simulink model "large_model"  (Simulink 8.0)
settings: Solver=ode45  StartTime=0.0  StopTime=10.0  RelTol=1e-3
(part 17 of 21)

== system large_model/RDC4002_1/RDC Hardware ==
  In : Inport
  PWR_In : Inport  Port=2
  Out : Outport
  wires:
  In/1 -> Out/1

== system large_model/RDC4002_2/RDC Hardware ==
  In : Inport
  PWR_In : Inport  Port=2
  Out : Outport
  wires:
  In/1 -> Out/1

== system large_model/RDC4002_3/RDC Hardware ==
  In : Inport
  PWR_In : Inport  Port=2
  Out : Outport
  wires:
  In/1 -> Out/1

== system large_model/RDC4002_4/RDC Hardware ==
  In : Inport
  PWR_In : Inport  Port=2
  Out : Outport
  wires:
  In/1 -> Out/1

== system large_model/GPM_IO_1/IO_Card/IO Card Hardware ==
  In : Inport
  PWR : Inport  Port=2
  Out : Outport
  wires:
  In/1 -> Out/1

== system large_model/GPM_IO_1/Integrated_GPM0/End_System ==
  From_GPM : Inport
  From_Switch_ChA : Inport  Port=2
  From_Switch_ChB : Inport  Port=3
  PWR : Inport  Port=4
  Bus Creator : BusCreator  Inputs=2
  ES Hardware : Subsystem
  To_GPM : Outport
  To_Switch_ChA : Outport  Port=2
  To_Switch_ChB : Outport  Port=3
  wires:
  From_GPM/1 -> ES Hardware/1(In1)
  Bus Creator/1 -> ES Hardware/2(In2)
  PWR/1 -> ES Hardware/3(PWR)
  ES Hardware/2(Out2) -> To_GPM/1
  ES Hardware/1(Out1) -> To_Switch_ChB/1, To_Switch_ChA/1
  From_Switch_ChB/1 -> Bus Creator/2  "Switch_ChB"
  From_Switch_ChA/1 -> Bus Creator/1  "Switch_ChA"

== system large_model/GPM_IO_1/Integrated_GPM0/GPM ==
  note: Este componente foi colocado devido a uma limitação do EFTG. Na versão 1.2.1,
      | não é permitido fazer uma conexão direta entre a entrada e a saída.
  From_IO : Inport
  PWR_In : Inport  Port=2
  GPM Hardware : Subsystem
  PWR : Subsystem
  To_ES : Outport
  PWR_Out : Outport  Port=2
  wires:
  PWR_In/1 -> GPM Hardware/2(PWR_In), PWR/1(In)
  GPM Hardware/1(Out) -> To_ES/1
  PWR/1(Out) -> PWR_Out/1
  From_IO/1 -> GPM Hardware/1(In)

== system large_model/GPM_IO_2/IO_Card/IO Card Hardware ==
  In : Inport
  PWR : Inport  Port=2
  Out : Outport
  wires:
  In/1 -> Out/1

== system large_model/GPM_IO_2/Integrated_GPM0/End_System ==
  From_GPM : Inport
  From_Switch_ChA : Inport  Port=2
  From_Switch_ChB : Inport  Port=3
  PWR : Inport  Port=4
  Bus Creator : BusCreator  Inputs=2
  ES Hardware : Subsystem
  To_GPM : Outport
  To_Switch_ChA : Outport  Port=2
  To_Switch_ChB : Outport  Port=3
  wires:
  From_GPM/1 -> ES Hardware/1(In1)
  Bus Creator/1 -> ES Hardware/2(In2)
  PWR/1 -> ES Hardware/3(PWR)
  ES Hardware/2(Out2) -> To_GPM/1
  ES Hardware/1(Out1) -> To_Switch_ChB/1, To_Switch_ChA/1
  From_Switch_ChB/1 -> Bus Creator/2  "Switch_ChB"
  From_Switch_ChA/1 -> Bus Creator/1  "Switch_ChA"

== system large_model/GPM_IO_2/Integrated_GPM0/GPM ==
  note: Este componente foi colocado devido a uma limitação do EFTG. Na versão 1.2.1,
      | não é permitido fazer uma conexão direta entre a entrada e a saída.
  From_IO : Inport
  PWR_In : Inport  Port=2
  GPM Hardware : Subsystem
  PWR : Subsystem
  To_ES : Outport
  PWR_Out : Outport  Port=2
  wires:
  PWR_In/1 -> GPM Hardware/2(PWR_In), PWR/1(In)
  GPM Hardware/1(Out) -> To_ES/1
  PWR/1(Out) -> PWR_Out/1
  From_IO/1 -> GPM Hardware/1(In)

== system large_model/GPM_IO_3/IO_Card/IO Card Hardware ==
  In : Inport
  PWR : Inport  Port=2
  Out : Outport
  wires:
  In/1 -> Out/1

== system large_model/GPM_IO_3/Integrated_GPM0/End_System ==
  From_GPM : Inport
  From_Switch_ChA : Inport  Port=2
  From_Switch_ChB : Inport  Port=3
  PWR : Inport  Port=4
  Bus Creator : BusCreator  Inputs=2
  ES Hardware : Subsystem
  To_GPM : Outport
  To_Switch_ChA : Outport  Port=2
  To_Switch_ChB : Outport  Port=3
  wires:
  From_GPM/1 -> ES Hardware/1(In1)
  Bus Creator/1 -> ES Hardware/2(In2)
  PWR/1 -> ES Hardware/3(PWR)
  ES Hardware/2(Out2) -> To_GPM/1
  ES Hardware/1(Out1) -> To_Switch_ChB/1, To_Switch_ChA/1
  From_Switch_ChB/1 -> Bus Creator/2  "Switch_ChB"
  From_Switch_ChA/1 -> Bus Creator/1  "Switch_ChA"

== system large_model/GPM_IO_3/Integrated_GPM0/GPM ==
  note: Este componente foi colocado devido a uma limitação do EFTG. Na versão 1.2.1,
      | não é permitido fazer uma conexão direta entre a entrada e a saída.
  From_IO : Inport
  PWR_In : Inport  Port=2
  GPM Hardware : Subsystem
  PWR : Subsystem
  To_ES : Outport
  PWR_Out : Outport  Port=2
  wires:
  PWR_In/1 -> GPM Hardware/2(PWR_In), PWR/1(In)
  GPM Hardware/1(Out) -> To_ES/1
  PWR/1(Out) -> PWR_Out/1
  From_IO/1 -> GPM Hardware/1(In)

== system large_model/GPM_IO_4/IO_Card/IO Card Hardware ==
  In : Inport
  PWR : Inport  Port=2
  Out : Outport
  wires:
  In/1 -> Out/1

== system large_model/GPM_IO_4/Integrated_GPM0/End_System ==
  From_GPM : Inport
  From_Switch_ChA : Inport  Port=2
  From_Switch_ChB : Inport  Port=3
  PWR : Inport  Port=4
  Bus Creator : BusCreator  Inputs=2
  ES Hardware : Subsystem
  To_GPM : Outport
  To_Switch_ChA : Outport  Port=2
  To_Switch_ChB : Outport  Port=3
  wires:
  From_GPM/1 -> ES Hardware/1(In1)
  Bus Creator/1 -> ES Hardware/2(In2)
  PWR/1 -> ES Hardware/3(PWR)
  ES Hardware/2(Out2) -> To_GPM/1
  ES Hardware/1(Out1) -> To_Switch_ChB/1, To_Switch_ChA/1
  From_Switch_ChB/1 -> Bus Creator/2  "Switch_ChB"
  From_Switch_ChA/1 -> Bus Creator/1  "Switch_ChA"

== system large_model/GPM_IO_4/Integrated_GPM0/GPM ==
  note: Este componente foi colocado devido a uma limitação do EFTG. Na versão 1.2.1,
      | não é permitido fazer uma conexão direta entre a entrada e a saída.
  From_IO : Inport
  PWR_In : Inport  Port=2
  GPM Hardware : Subsystem
  PWR : Subsystem
  To_ES : Outport
  PWR_Out : Outport  Port=2
  wires:
  PWR_In/1 -> GPM Hardware/2(PWR_In), PWR/1(In)
  GPM Hardware/1(Out) -> To_ES/1
  PWR/1(Out) -> PWR_Out/1
  From_IO/1 -> GPM Hardware/1(In)

== system large_model/Integrated_GPM1_1/End_System/ES Hardware ==
  In1 : Inport
  In2 : Inport  Port=2
  PWR : Inport  Port=3
  Out1 : Outport
  Out2 : Outport  Port=2
  wires:
  In1/1 -> Out1/1
  In2/1 -> Out2/1

== system large_model/Integrated_GPM1_1/GPM/CAB1_FCC1 ==
  PedalYaw1_cmd : Inport
  PedalYaw2_cmd : Inport  Port=2
  PedalYaw3_cmd : Inport  Port=3
  PedalYaw4_cmd : Inport  Port=4
  in_sup : Inport  Port=5
  yaw_GPM2_cmd : Inport  Port=6
  REU_LVDT_AIL_LIB_dat : Inport  Port=7
  REU_mon_AIL_LIB_dat : Inport  Port=8
  REU_LVDT_AIL_LOB_dat : Inport  Port=9
  ads_dat : Inport  Port=10
  REU_AIL_LVDT_RIB_dat : Inport  Port=11
  REU_AIL_mon_RIB_dat : Inport  Port=12
  REU_AIL_LVDT_ROB_dat : Inport  Port=13
  REU_AIL_mon_ROB_dat : Inport  Port=14
  sidestick1Pitch1_cmd : Inport  Port=15
  sidestick1Pitch2_cmd : Inport  Port=16
  sidestick1Pitch3_cmd : Inport  Port=17
  sidestick1Pitch4_cmd : Inport  Port=18
  sidestick1Roll1_cmd : Inport  Port=19
  sidestick1Roll2_cmd : Inport  Port=20
  sidestick1Roll3_cmd : Inport  Port=21
  sidestick1Roll4_cmd : Inport  Port=22
  sidestick2Pitch1_cmd : Inport  Port=23
  sidestick2Pitch2_cmd : Inport  Port=24
  sidestick2Pitch3_cmd : Inport  Port=25
  sidestick2Pitch4_cmd : Inport  Port=26
  sidestick2Roll1_cmd : Inport  Port=27
  sidestick2Roll2_cmd : Inport  Port=28
  sidestick2Roll3_cmd : Inport  Port=29
  sidestick2Roll4_cmd : Inport  Port=30
  pitch_roll_GPM2_cmd : Inport  Port=31
  REU_ELEV_LVDT_LIB_dat : Inport  Port=32
  REU_ELEV_mon_LIB_dat : Inport  Port=33
  REU_ELEV_LVDT_LOB_dat : Inport  Port=34
  REU_ELEV_mon_LOB_dat : Inport  Port=35
  REU1_LVDT_rudder_dat : Inport  Port=36
  REU1_MON_rudder_dat : Inport  Port=37
  REU2_LVDT_rudder_dat : Inport  Port=38
  REU2_MON_rudder_dat : Inport  Port=39
  REU_mon_AIL_LOB_dat : Inport  Port=40
  REU_ELEV_LVDT_RIB_dat : Inport  Port=41
  REU_ELEV_mon_RIB_dat : Inport  Port=42
  REU_ELEV_LVDT_ROB_dat : Inport  Port=43
  REU_ELEV_mon_ROB_dat : Inport  Port=44
  fcc_ram_mon_rudder_LWR_GPM2_dat : Inport  Port=45
  fcc_ram_mon_rudder_UPR_GPM2_dat : Inport  Port=46
  fcc_ram_mon_AIL_LIB_GPM2_dat : Inport  Port=47
  fcc_ram_mon_AIL_LOB_GPM2_dat : Inport  Port=48
  fcc_ram_mon_AIL_RIB_GPM2_dat : Inport  Port=49
  fcc_ram_mon_AIL_ROB_GPM2_dat : Inport  Port=50
  fcc_ram_mon_ELEV_LIB_GPM2_dat : Inport  Port=51
  fcc_ram_mon_ELEV_LOB_GPM2_dat : Inport  Port=52
  fcc_ram_mon_ELEV_RIB_GPM2_da : Inport  Port=53
  fcc_ram_mon_ELEV_ROB_GPM2_dat : Inport  Port=54
  Yaw : Subsystem
  yaw_cmd : Outport
  pitch_roll_cmd : Outport  Port=2
  fcc_ram_mon_rudder_LWR_dat : Outport  Port=3
  fcc_ram_mon_rudder_UPR_dat : Outport  Port=4
  fcc_ram_mon_AIL_LIB_dat : Outport  Port=5
  fcc_ram_mon_AIL_LOB_dat : Outport  Port=6
  fcc_ram_mon_AIL_RIB_dat : Outport  Port=7
  fcc_ram_mon_AIL_ROB_dat : Outport  Port=8
  fcc_ram_mon_ELEV_LIB_dat : Outport  Port=9
  fcc_ram_mon_ELEV_LOB_dat : Outport  Port=10
  fcc_ram_mon_ELEV_RIB_dat : Outport  Port=11
  fcc_ram_mon_ELEV_ROB_dat : Outport  Port=12
  wires:
  Yaw/21(fcc_ram_mon_rudder_UPR_dat) -> fcc_ram_mon_rudder_UPR_dat/1
  fcc_ram_mon_ELEV_ROB_GPM2_dat/1 -> Yaw/63(fcc_ram_mon_ELEV_ROB_GPM2_dat)
  fcc_ram_mon_ELEV_RIB_GPM2_da/1 -> Yaw/62(fcc_ram_mon_ELEV_RIB_GPM2_dat)
  fcc_ram_mon_ELEV_LOB_GPM2_dat/1 -> Yaw/61(fcc_ram_mon_ELEV_LOB_GPM2_dat)
  fcc_ram_mon_AIL_ROB_GPM2_dat/1 -> Yaw/60(fcc_ram_mon_AIL_ROB_GPM2_dat)
  fcc_ram_mon_AIL_RIB_GPM2_dat/1 -> Yaw/59(fcc_ram_mon_AIL_RIB_GPM2_dat)
  fcc_ram_mon_AIL_LOB_GPM2_dat/1 -> Yaw/58(fcc_ram_mon_AIL_LOB_GPM2_dat)
  fcc_ram_mon_rudder_UPR_GPM2_dat/1 -> Yaw/57(fcc_ram_mon_rudder_UPR_GPM2_dat)
  Yaw/20(fcc_ram_mon_ELEV_ROB_dat) -> fcc_ram_mon_ELEV_ROB_dat/1
  Yaw/19(fcc_ram_mon_ELEV_RIB_dat) -> fcc_ram_mon_ELEV_RIB_dat/1
  Yaw/18(fcc_ram_mon_ELEV_LOB_dat) -> fcc_ram_mon_ELEV_LOB_dat/1
  Yaw/17(fcc_ram_mon_ELEV_LIB_dat) -> fcc_ram_mon_ELEV_LIB_dat/1
  fcc_ram_mon_ELEV_LIB_GPM2_dat/1 -> Yaw/56(fcc_ram_mon_ELEV_LIB_GPM2_dat)
  REU_ELEV_LVDT_RIB_dat/1 -> Yaw/52(REU_ELEV_LVDT_RIB_dat)
  REU_ELEV_mon_ROB_dat/1 -> Yaw/55(REU_ELEV_mon_ROB_dat)
  REU_ELEV_LVDT_ROB_dat/1 -> Yaw/54(REU_ELEV_LVDT_ROB_dat)
  REU_ELEV_mon_RIB_dat/1 -> Yaw/53(REU_ELEV_mon_RIB_dat)
  Yaw/16(fcc_ram_mon_AIL_ROB_dat) -> fcc_ram_mon_AIL_ROB_dat/1
  Yaw/15(fcc_ram_mon_AIL_RIB_dat) -> fcc_ram_mon_AIL_RIB_dat/1
  Yaw/14(fcc_ram_mon_AIL_LOB_dat) -> fcc_ram_mon_AIL_LOB_dat/1
  Yaw/13(fcc_ram_mon_AIL_LIB_dat) -> fcc_ram_mon_AIL_LIB_dat/1
  fcc_ram_mon_AIL_LIB_GPM2_dat/1 -> Yaw/51(fcc_ram_mon_AIL_LIB_GPM2_dat)
  REU_mon_AIL_LOB_dat/1 -> Yaw/50(REU_mon_AIL_LOB_dat)
  REU_LVDT_AIL_LOB_dat/1 -> Yaw/9(REU_LVDT_AIL_LOB_dat)
  REU_mon_AIL_LIB_dat/1 -> Yaw/8(REU_mon_AIL_LIB_dat)
  REU_LVDT_AIL_LIB_dat/1 -> Yaw/7(REU_LVDT_AIL_LIB_dat)
  REU2_MON_rudder_dat/1 -> Yaw/49(REU2_MON_rudder_dat)
  REU2_LVDT_rudder_dat/1 -> Yaw/48(REU2_LVDT_rudder_dat)
  REU1_MON_rudder_dat/1 -> Yaw/47(REU1_MON_rudder_dat)
  REU1_LVDT_rudder_dat/1 -> Yaw/46(REU1_LVDT_rudder_dat)
  REU_ELEV_mon_LOB_dat/1 -> Yaw/45(REU_ELEV_mon_LOB_dat)
  REU_ELEV_LVDT_LOB_dat/1 -> Yaw/44(REU_ELEV_LVDT_LOB_dat)
  REU_ELEV_mon_LIB_dat/1 -> Yaw/43(REU_ELEV_mon_LIB_dat)
  REU_ELEV_LVDT_LIB_dat/1 -> Yaw/42(REU_ELEV_LVDT_LIB_dat)
  pitch_roll_GPM2_cmd/1 -> Yaw/41(pitch_roll_GPM2_cmd)
  Yaw/12(pitch_roll_cmd) -> pitch_roll_cmd/1
  sidestick2Roll4_cmd/1 -> Yaw/40(sidestick2Roll4_cmd)
  sidestick2Roll3_cmd/1 -> Yaw/39(sidestick2Roll3_cmd)
  sidestick2Roll2_cmd/1 -> Yaw/38(sidestick2Roll2_cmd)
  sidestick2Roll1_cmd/1 -> Yaw/37(sidestick2Roll1_cmd)
  sidestick2Pitch4_cmd/1 -> Yaw/36(sidestick2Pitch4_cmd)
  sidestick2Pitch3_cmd/1 -> Yaw/35(sidestick2Pitch3_cmd)
  sidestick2Pitch2_cmd/1 -> Yaw/34(sidestick2Pitch2_cmd)
  sidestick2Pitch1_cmd/1 -> Yaw/33(sidestick2Pitch1_cmd)
  Yaw/10(out_sup) -> Yaw/20(in_sup)
  sidestick1Roll4_cmd/1 -> Yaw/32(sidestick1Roll4_cmd)
  sidestick1Roll3_cmd/1 -> Yaw/31(sidestick1Roll3_cmd)
  sidestick1Roll2_cmd/1 -> Yaw/30(sidestick1Roll2_cmd)
  sidestick1Roll1_cmd/1 -> Yaw/29(sidestick1Roll1_cmd)
  sidestick1Pitch4_cmd/1 -> Yaw/28(sidestick1Pitch4_cmd)
  sidestick1Pitch3_cmd/1 -> Yaw/27(sidestick1Pitch3_cmd)
  sidestick1Pitch2_cmd/1 -> Yaw/26(sidestick1Pitch2_cmd)
  sidestick1Pitch1_cmd/1 -> Yaw/25(sidestick1Pitch1_cmd)
  REU_AIL_mon_ROB_dat/1 -> Yaw/24(REU_AIL_mon_ROB_dat)
  REU_AIL_LVDT_ROB_dat/1 -> Yaw/23(REU_AIL_LVDT_ROB_dat)
  REU_AIL_mon_RIB_dat/1 -> Yaw/22(REU_AIL_mon_RIB_dat)
  REU_AIL_LVDT_RIB_dat/1 -> Yaw/21(REU_AIL_LVDT_RIB_dat)
  fcc_ram_mon_rudder_LWR_GPM2_dat/1 -> Yaw/19(fcc_ram_mon_rudder_LWR_GPM2_dat)
  Yaw/11(fcc_ram_mon_rudder_LWR_dat) -> fcc_ram_mon_rudder_LWR_dat/1
  yaw_GPM2_cmd/1 -> Yaw/6(yaw_GPM2_cmd)
  in_sup/1 -> Yaw/5(Pedal_MechanicalFailure_cmd)
  PedalYaw4_cmd/1 -> Yaw/4(PedalYaw4_cmd)
  PedalYaw3_cmd/1 -> Yaw/3(PedalYaw3_cmd)
  PedalYaw2_cmd/1 -> Yaw/2(PedalYaw2_cmd)
  Yaw/1(yaw_cmd) -> yaw_cmd/1
  PedalYaw1_cmd/1 -> Yaw/1(PedalYaw1_cmd)
  [pedal_failures] -> Yaw/11(pedal_dat)
  Yaw/2(pedal_out) -> [pedal_failures]
  Yaw/3(pedal_loss_3_lvdt_out) -> [pedal_loss_3_lvdt]
  [pedal_loss_3_lvdt] -> Yaw/12(pedal_loss_3_lvdt_dat)
  [pedal_err_3_lvdt] -> Yaw/13(pedal_erroneous_3_lvdt_dat)
  Yaw/4(pedal_erroneous_3_lvdt_out) -> [pedal_err_3_lvdt]
  Yaw/5(loss_3_lvdt_lvdt4_erroneous_out) -> [loss_3_lvdt_lvdt4_err]
  Yaw/6(loss_3_lvdt_lvdt3_erroneous_out) -> [loss_3_lvdt_lvdt3_err]
  Yaw/7(loss_3_lvdt_lvdt2_erroneous_out) -> [loss_3_lvdt_lvdt2_err]
  Yaw/8(loss_3_lvdt_lvdt1_erroneous_out) -> [loss_3_lvdt_lvdt1_err]
  [loss_3_lvdt_lvdt4_err] -> Yaw/14(loss_3_lvdt_lvdt4_erroneous_dat)
  [loss_3_lvdt_lvdt3_err] -> Yaw/15(loss_3_lvdt_lvdt3_erroneous_dat)
  [loss_3_lvdt_lvdt2_err] -> Yaw/16(loss_3_lvdt_lvdt2_erroneous_dat)
  [loss_3_lvdt_lvdt1_err] -> Yaw/17(loss_3_lvdt_lvdt1_erroneous_dat)
  ads_dat/1 -> Yaw/10(ads_dat)
  Yaw/9(loss_3_lvdt_lvdt_1_erroneous_out) -> [loss_3_lvdt_lvdt_1_err]
  [loss_3_lvdt_lvdt_1_err] -> Yaw/18(loss_3_lvdt_lvdt_1_erroneous_dat)

== system large_model/Integrated_GPM1_1/GPM/GPM Hardware ==
  Data : Inport
  PWR_In : Inport  Port=2
  Out : Outport
  wires:
  Data/1 -> Out/1

== system large_model/Integrated_GPM1_1/GPM/PWR ==
  In1 : Inport
  Out1 : Outport
  wires:
  In1/1 -> Out1/1

== system large_model/Integrated_GPM1_1/GPM/SCP_CAB1_GPM2_fcc_ram_mon_AIL_LIB ==
  In1 : Inport
  In2 : Inport  Port=2
  Out : Outport

== system large_model/Integrated_GPM1_1/GPM/SCP_CAB1_GPM2_fcc_ram_mon_AIL_LOB ==
  In1 : Inport
  In2 : Inport  Port=2
  Out : Outport

== system large_model/Integrated_GPM1_1/GPM/SCP_CAB1_GPM2_fcc_ram_mon_AIL_RIB ==
  In1 : Inport
  In2 : Inport  Port=2
  Out : Outport

== system large_model/Integrated_GPM1_1/GPM/SCP_CAB1_GPM2_fcc_ram_mon_AIL_ROB ==
  In1 : Inport
  In2 : Inport  Port=2
  Out : Outport

== system large_model/Integrated_GPM1_1/GPM/SCP_CAB1_GPM2_fcc_ram_mon_ELEV_LIB ==
  In1 : Inport
  In2 : Inport  Port=2
  Out : Outport

== system large_model/Integrated_GPM1_1/GPM/SCP_CAB1_GPM2_fcc_ram_mon_ELEV_LOB ==
  In1 : Inport
  In2 : Inport  Port=2
  Out : Outport

== system large_model/Integrated_GPM1_1/GPM/SCP_CAB1_GPM2_fcc_ram_mon_ELEV_RIB ==
  In1 : Inport
  In2 : Inport  Port=2
  Out : Outport

== system large_model/Integrated_GPM1_1/GPM/SCP_CAB1_GPM2_fcc_ram_mon_ELEV_ROB ==
  In1 : Inport
  In2 : Inport  Port=2
  Out : Outport

== system large_model/Integrated_GPM1_1/GPM/SCP_CAB1_GPM2_fcc_ram_mon_rudder_LWR ==
  In1 : Inport
  In2 : Inport  Port=2
  Out : Outport

== system large_model/Integrated_GPM1_1/GPM/SCP_CAB1_GPM2_fcc_ram_mon_rudder_UPR ==
  In1 : Inport
  In2 : Inport  Port=2
  Out : Outport

== system large_model/Integrated_GPM1_1/GPM/SCP_CAB1_GPM2_pitch_roll ==
  In1 : Inport
  In2 : Inport  Port=2
  Out : Outport

== system large_model/Integrated_GPM1_1/GPM/SCP_CAB1_GPM2_yaw ==
  In1 : Inport
  In2 : Inport  Port=2
  Out : Outport

== system large_model/Integrated_GPM1_1/GPM/SCP_ail_REU_LIB_LVDT ==
  In1 : Inport
  In2 : Inport  Port=2
  Out : Outport

== system large_model/Integrated_GPM1_1/GPM/SCP_ail_REU_LIB_mon ==
  In1 : Inport
  In2 : Inport  Port=2
  Out : Outport

== system large_model/Integrated_GPM1_1/GPM/SCP_ail_REU_LOB_LVDT ==
  In1 : Inport
  In2 : Inport  Port=2
  Out : Outport

== system large_model/Integrated_GPM1_1/GPM/SCP_ail_REU_LOB_mon ==
  In1 : Inport
  In2 : Inport  Port=2
  Out : Outport

== system large_model/Integrated_GPM1_1/GPM/SCP_ail_REU_RIB_LVDT ==
  In1 : Inport
  In2 : Inport  Port=2
  Out : Outport

== system large_model/Integrated_GPM1_1/GPM/SCP_ail_REU_RIB_mon ==
  In1 : Inport
  In2 : Inport  Port=2
  Out : Outport

== system large_model/Integrated_GPM1_1/GPM/SCP_ail_REU_ROB_LVDT ==
  In1 : Inport
  In2 : Inport  Port=2
  Out : Outport

== system large_model/Integrated_GPM1_1/GPM/SCP_ail_REU_ROB_mon ==
  In1 : Inport
  In2 : Inport  Port=2
  Out : Outport

== system large_model/Integrated_GPM1_1/GPM/SCP_elev_REU_LIB_LVDT ==
  In1 : Inport
  In2 : Inport  Port=2
  Out : Outport

== system large_model/Integrated_GPM1_1/GPM/SCP_elev_REU_LIB_mon_dat ==
  In1 : Inport
  In2 : Inport  Port=2
  Out : Outport

== system large_model/Integrated_GPM1_1/GPM/SCP_elev_REU_LOB_LVDT ==
  In1 : Inport
  In2 : Inport  Port=2
  Out : Outport

== system large_model/Integrated_GPM1_1/GPM/SCP_elev_REU_LOB_mon ==
  In1 : Inport
  In2 : Inport  Port=2
  Out : Outport

== system large_model/Integrated_GPM1_1/GPM/SCP_elev_REU_RIB_LVDT ==
  In1 : Inport
  In2 : Inport  Port=2
  Out : Outport

== system large_model/Integrated_GPM1_1/GPM/SCP_elev_REU_RIB_mon ==
  In1 : Inport
  In2 : Inport  Port=2
  Out : Outport

== system large_model/Integrated_GPM1_1/GPM/SCP_elev_REU_ROB_LVDT ==
  In1 : Inport
  In2 : Inport  Port=2
  Out : Outport

== system large_model/Integrated_GPM1_1/GPM/SCP_elev_REU_ROB_mon ==
  In1 : Inport
  In2 : Inport  Port=2
  Out : Outport

== system large_model/Integrated_GPM1_1/GPM/SCP_rud_REU1_LVDT ==
  In1 : Inport
  In2 : Inport  Port=2
  Out : Outport

== system large_model/Integrated_GPM1_1/GPM/SCP_rud_REU1_MON ==
  In1 : Inport
  In2 : Inport  Port=2
  Out : Outport

== system large_model/Integrated_GPM1_1/GPM/SCP_rud_REU2_LVDT ==
  In1 : Inport
  In2 : Inport  Port=2
  Out : Outport

== system large_model/Integrated_GPM1_1/GPM/SCP_rud_REU2_MON ==
  In1 : Inport
  In2 : Inport  Port=2
  Out : Outport

== system large_model/Integrated_GPM1_1/GPM/Self_checking_pair_ADS1A ==
  In1 : Inport
  In2 : Inport  Port=2
  In3 : Inport  Port=3
  In4 : Inport  Port=4
  In5 : Inport  Port=5
  In6 : Inport  Port=6
  In7 : Inport  Port=7
  In8 : Inport  Port=8
  Out : Outport
  wires:
  In1/1 -> Out/1

== system large_model/Integrated_GPM1_1/GPM/Self_checking_pair_ADS1B ==
  In1 : Inport
  In2 : Inport  Port=2
  In3 : Inport  Port=3
  In4 : Inport  Port=4
  In5 : Inport  Port=5
  In6 : Inport  Port=6
  In7 : Inport  Port=7
  In8 : Inport  Port=8
  Out : Outport
  wires:
  In1/1 -> Out/1

== system large_model/Integrated_GPM1_1/GPM/Self_checking_pair_ADS2A ==
  In1 : Inport
  In2 : Inport  Port=2
  In3 : Inport  Port=3
  In4 : Inport  Port=4
  In5 : Inport  Port=5
  In6 : Inport  Port=6
  In7 : Inport  Port=7
  In8 : Inport  Port=8
  Out : Outport
  wires:
  In1/1 -> Out/1

== system large_model/Integrated_GPM1_1/GPM/Self_checking_pair_ADS2B ==
  In1 : Inport
  In2 : Inport  Port=2
  In3 : Inport  Port=3
  In4 : Inport  Port=4
  In5 : Inport  Port=5
  In6 : Inport  Port=6
  In7 : Inport  Port=7
  In8 : Inport  Port=8
  Out : Outport
  wires:
  In1/1 -> Out/1

== system large_model/Integrated_GPM1_1/GPM/Self_checking_pair_ADS3A ==
  In1 : Inport
  In2 : Inport  Port=2
  In3 : Inport  Port=3
  In4 : Inport  Port=4
  In5 : Inport  Port=5
  In6 : Inport  Port=6
  In7 : Inport  Port=7
  In8 : Inport  Port=8
  Out : Outport
  wires:
  In1/1 -> Out/1

== system large_model/Integrated_GPM1_1/GPM/Self_checking_pair_ADS3B ==
  In1 : Inport
  In2 : Inport  Port=2
  In3 : Inport  Port=3
  In4 : Inport  Port=4
  In5 : Inport  Port=5
  In6 : Inport  Port=6
  In7 : Inport  Port=7
  In8 : Inport  Port=8
  Out : Outport
  wires:
  In1/1 -> Out/1

== system large_model/Integrated_GPM1_1/GPM/Self_checking_pair_ADS4A ==
  In1 : Inport
  In2 : Inport  Port=2
  In3 : Inport  Port=3
  In4 : Inport  Port=4
  In5 : Inport  Port=5
  In6 : Inport  Port=6
  In7 : Inport  Port=7
  In8 : Inport  Port=8
  Out : Outport
  wires:
  In1/1 -> Out/1

== system large_model/Integrated_GPM1_1/GPM/Self_checking_pair_ADS4B ==
  In1 : Inport
  In2 : Inport  Port=2
  In3 : Inport  Port=3
  In4 : Inport  Port=4
  In5 : Inport  Port=5
  In6 : Inport  Port=6
  In7 : Inport  Port=7
  In8 : Inport  Port=8
  Out : Outport
  wires:
  In1/1 -> Out/1

== system large_model/Integrated_GPM1_1/GPM/Self_checking_pair_PedalYaw1 ==
  In1 : Inport
  In2 : Inport  Port=2
  In3 : Inport  Port=3
  In4 : Inport  Port=4
  In5 : Inport  Port=5
  In6 : Inport  Port=6
  In7 : Inport  Port=7
  In8 : Inport  Port=8
  Out : Outport
  wires:
  In1/1 -> Out/1

== system large_model/Integrated_GPM1_1/GPM/Self_checking_pair_PedalYaw2 ==
  In1 : Inport
  In2 : Inport  Port=2
  In3 : Inport  Port=3
  In4 : Inport  Port=4
  In5 : Inport  Port=5
  In6 : Inport  Port=6
  In7 : Inport  Port=7
  In8 : Inport  Port=8
  Out : Outport
  wires:
  In1/1 -> Out/1

== system large_model/Integrated_GPM1_1/GPM/Self_checking_pair_PedalYaw3 ==
  In1 : Inport
  In2 : Inport  Port=2
  In3 : Inport  Port=3
  In4 : Inport  Port=4
  In5 : Inport  Port=5
  In6 : Inport  Port=6
  In7 : Inport  Port=7
  In8 : Inport  Port=8
  Out : Outport
  wires:
  In1/1 -> Out/1

== system large_model/Integrated_GPM1_1/GPM/Self_checking_pair_PedalYaw4 ==
  In1 : Inport
  In2 : Inport  Port=2
  In3 : Inport  Port=3
  In4 : Inport  Port=4
  In5 : Inport  Port=5
  In6 : Inport  Port=6
  In7 : Inport  Port=7
  In8 : Inport  Port=8
  Out : Outport
  wires:
  In1/1 -> Out/1

== system large_model/Integrated_GPM1_1/GPM/Self_checking_pair_SSC1_pitch1 ==
  In1 : Inport
  In2 : Inport  Port=2
  In3 : Inport  Port=3
  In4 : Inport  Port=4
  In5 : Inport  Port=5
  In6 : Inport  Port=6
  In7 : Inport  Port=7
  In8 : Inport  Port=8
  Out : Outport
  wires:
  In1/1 -> Out/1

== system large_model/Integrated_GPM1_1/GPM/Self_checking_pair_SSC1_pitch2 ==
  In1 : Inport
  In2 : Inport  Port=2
  In3 : Inport  Port=3
  In4 : Inport  Port=4
  In5 : Inport  Port=5
  In6 : Inport  Port=6
  In7 : Inport  Port=7
  In8 : Inport  Port=8
  Out : Outport
  wires:
  In1/1 -> Out/1

== system large_model/Integrated_GPM1_1/GPM/Self_checking_pair_SSC1_pitch3 ==
  In1 : Inport
  In2 : Inport  Port=2
  In3 : Inport  Port=3
  In4 : Inport  Port=4
  In5 : Inport  Port=5
  In6 : Inport  Port=6
  In7 : Inport  Port=7
  In8 : Inport  Port=8
  Out : Outport
  wires:
  In1/1 -> Out/1

== system large_model/Integrated_GPM1_1/GPM/Self_checking_pair_SSC1_pitch4 ==
  In1 : Inport
  In2 : Inport  Port=2
  In3 : Inport  Port=3
  In4 : Inport  Port=4
  In5 : Inport  Port=5
  In6 : Inport  Port=6
  In7 : Inport  Port=7
  In8 : Inport  Port=8
  Out : Outport
  wires:
  In1/1 -> Out/1

== system large_model/Integrated_GPM1_1/GPM/Self_checking_pair_SSC1_roll1 ==
  In1 : Inport
  In2 : Inport  Port=2
  In3 : Inport  Port=3
  In4 : Inport  Port=4
  In5 : Inport  Port=5
  In6 : Inport  Port=6
  In7 : Inport  Port=7
  In8 : Inport  Port=8
  Out : Outport
  wires:
  In1/1 -> Out/1

== system large_model/Integrated_GPM1_1/GPM/Self_checking_pair_SSC1_roll2 ==
  In1 : Inport
  In2 : Inport  Port=2
  In3 : Inport  Port=3
  In4 : Inport  Port=4
  In5 : Inport  Port=5
  In6 : Inport  Port=6
  In7 : Inport  Port=7
  In8 : Inport  Port=8
  Out : Outport
  wires:
  In1/1 -> Out/1

== system large_model/Integrated_GPM1_1/GPM/Self_checking_pair_SSC1_roll3 ==
  In1 : Inport
  In2 : Inport  Port=2
  In3 : Inport  Port=3
  In4 : Inport  Port=4
  In5 : Inport  Port=5
  In6 : Inport  Port=6
  In7 : Inport  Port=7
  In8 : Inport  Port=8
  Out : Outport
  wires:
  In1/1 -> Out/1

== system large_model/Integrated_GPM1_1/GPM/Self_checking_pair_SSC1_roll4 ==
  In1 : Inport
  In2 : Inport  Port=2
  In3 : Inport  Port=3
  In4 : Inport  Port=4
  In5 : Inport  Port=5
  In6 : Inport  Port=6
  In7 : Inport  Port=7
  In8 : Inport  Port=8
  Out : Outport
  wires:
  In1/1 -> Out/1

== system large_model/Integrated_GPM1_1/GPM/Self_checking_pair_SSC2_pitch1 ==
  In1 : Inport
  In2 : Inport  Port=2
  In3 : Inport  Port=3
  In4 : Inport  Port=4
  In5 : Inport  Port=5
  In6 : Inport  Port=6
  In7 : Inport  Port=7
  In8 : Inport  Port=8
  Out : Outport
  wires:
  In1/1 -> Out/1

== system large_model/Integrated_GPM1_1/GPM/Self_checking_pair_SSC2_pitch2 ==
  In1 : Inport
  In2 : Inport  Port=2
  In3 : Inport  Port=3
  In4 : Inport  Port=4
  In5 : Inport  Port=5
  In6 : Inport  Port=6
  In7 : Inport  Port=7
  In8 : Inport  Port=8
  Out : Outport
  wires:
  In1/1 -> Out/1
